simulink model "controller_observer_AllAgents_v5"  (Simulink 8.4)
settings: Solver=ode1  StartTime=0.0  StopTime=inf  FixedStep=60*1  RelTol=1e-3
values from neighbouring files (symbols this model uses):
  Cpw = 4180    [readepJSONout.m]
  m_max = 3    [DesignParameters.m]
  mc_MAX = 2    [DesignParameters.m]
  Tamb_additive_Sensor_fault_time = 60*60*24*(Tamb_day_ofthe_add_fault + Tamb_time_ofthe_add_fault)    (= 129600)    [faults_setting.m]
  Tamb_additive_Sensor_fault_value = 10    [faults_setting.m]
  Tamb_mult_Sensor_fault_time = 60*60*24*(Tamb_day_ofthe_mult_fault + Tamb_time_ofthe_mult_fault)    (= 129600)    [faults_setting.m]
  Tamb_mult_Sensor_fault_value = 1.1    [faults_setting.m]
  Ts = 3600/dat.Timestep.Timestep_0x20_1.number_of_timesteps_per_hour    [readepJSONout.m]
  Tst_C_additive_Sensor_fault_time = 60*60*24*(Tst_C_day_ofthe_add_fault + Tst_C_time_ofthe_add_fault)    (= 129600)    [faults_setting.m]
  Tst_C_additive_Sensor_fault_value = 10    [faults_setting.m]
  Tst_C_mult_Sensor_fault_time = 60*60*24*(Tst_C_day_ofthe_mult_fault + Tst_C_time_ofthe_mult_fault)    (= 129600)    [faults_setting.m]
  Tst_C_mult_Sensor_fault_value = 1.1    [faults_setting.m]
  Tst_H_additive_Sensor_fault_time = 60*60*24*(Tst_H_day_ofthe_add_fault + Tst_H_time_ofthe_add_fault)    (= 129600)    [faults_setting.m]
  Tst_H_additive_Sensor_fault_value = 10    [faults_setting.m]
  Tst_H_mult_Sensor_fault_time = 60*60*24*(Tst_H_day_ofthe_mult_fault + Tst_H_time_ofthe_mult_fault)    (= 129600)    [faults_setting.m]
  Tst_H_mult_Sensor_fault_value = 1.1    [faults_setting.m]
undefined symbols (not defined in the file or in its folder): building, COP, Ehp_max, fsa_E, fsa_ET, fsa_N, fsa_NT, fsa_W, fsa_WT, h_E, h_ET, h_N, h_NT, h_W, h_WT, K_NtoNT, K_NTtoN, Ke_E, Ke_ET, Ke_N, Ke_NT, Ke_WT, Km_E, Km_ET, Km_N, Km_NT, Km_W, Km_WT, Kout_N, Kout_NT, Kout_W, Kout_WT, Kst, Ksta, Kstcij, KstRef, Kw_E, Kw_ET, Kw_N, Kw_NT, Kw_W, Kw_WT
libraries: simulink x61
(part 16 of 36)

== system controller_observer_AllAgents_v5/Controller/Fault_mc_MainCorridor_Cooling ==
  In : Inport
  Add : Sum  Inputs=+++  SampleTime=Ts
  Add2 : Sum  Inputs=+-  SampleTime=Ts
  Clock : Clock
  Constant : Constant  Value=building.zone(17).mc_C_mult_Sensor_fault_time  SampleTime=Ts
  Constant2 : Constant  Value=building.zone(17).mc_C_incr_Sensor_fault_time  SampleTime=Ts
  Dot Product : DotProduct  SampleTime=Ts  InputSameDT=on  OutDataTypeStr=Inherit: Inherit via internal rule
  Fcn : Fcn  Expr=1-0.99^(u)  SampleTime=Ts
  Gain : Gain  Gain=building.zone(17).mc_C_mult_Sensor_fault_value  SampleTime=Ts
  Gain1 : Gain  Gain=building.zone(17).mc_C_incr_Sensor_fault_value  SampleTime=Ts
  Gain3 : Gain  Gain=1/Ts  SampleTime=Ts
  If : If  NumInputs=2  IfExpression=u1 >= u2  SampleTime=Ts
  If Action Subsystem : Subsystem  TreatAsAtomicUnit=on
  If Action Subsystem1 : Subsystem  TreatAsAtomicUnit=on
  Merge : Merge
  Step : Step  Time=building.zone(17).mc_C_additive_Sensor_fault_time  After=building.zone(17).mc_C_additive_Sensor_fault_value  SampleTime=Ts
  Step1 : Step  Time=building.zone(17).mc_C_incr_Sensor_fault_time  SampleTime=Ts
  Out : Outport
  wires:
  If/2 -> If Action Subsystem1/Action
  Add/1 -> Out/1
  Step/1 -> Add/1
  Merge/1 -> Add/2
  If/1 -> If Action Subsystem/Action
  If Action Subsystem/1(Out1) -> Merge/1
  In/1 -> If Action Subsystem1/1(In1), Gain/1
  If Action Subsystem1/1(Out1) -> Merge/2
  Gain/1 -> If Action Subsystem/1(In1)
  Constant/1 -> If/2
  Clock/1 -> Add2/1, If/1  "k"
  Dot Product/1 -> Fcn/1
  Step1/1 -> Dot Product/2
  Constant2/1 -> Add2/2
  Fcn/1 -> Gain1/1
  Add2/1 -> Gain3/1
  Gain3/1 -> Dot Product/1
  Gain1/1 -> Add/3

== system controller_observer_AllAgents_v5/Controller/Fault_mc_MainCorridor_Heating ==
  In : Inport
  Add : Sum  Inputs=+++  SampleTime=Ts
  Add2 : Sum  Inputs=+-  SampleTime=Ts
  Clock : Clock
  Constant : Constant  Value=building.zone(17).mc_H_mult_Sensor_fault_time  SampleTime=Ts
  Constant2 : Constant  Value=building.zone(17).mc_H_incr_Sensor_fault_time  SampleTime=Ts
  Dot Product : DotProduct  SampleTime=Ts  InputSameDT=on  OutDataTypeStr=Inherit: Inherit via internal rule
  Fcn : Fcn  Expr=1-0.99^(u)  SampleTime=Ts
  Gain : Gain  Gain=building.zone(17).mc_H_mult_Sensor_fault_value  SampleTime=Ts
  Gain1 : Gain  Gain=building.zone(17).mc_H_incr_Sensor_fault_value  SampleTime=Ts
  Gain3 : Gain  Gain=1/Ts  SampleTime=Ts
  If : If  NumInputs=2  IfExpression=u1 >= u2  SampleTime=Ts
  If Action Subsystem : Subsystem  TreatAsAtomicUnit=on
  If Action Subsystem1 : Subsystem  TreatAsAtomicUnit=on
  Merge : Merge
  Step : Step  Time=building.zone(17).mc_H_additive_Sensor_fault_time  After=building.zone(17).mc_H_additive_Sensor_fault_value  SampleTime=Ts
  Step1 : Step  Time=building.zone(17).mc_H_incr_Sensor_fault_time  SampleTime=Ts
  Out : Outport
  wires:
  If/2 -> If Action Subsystem1/Action
  Add/1 -> Out/1
  Step/1 -> Add/1
  Merge/1 -> Add/2
  If/1 -> If Action Subsystem/Action
  If Action Subsystem/1(Out1) -> Merge/1
  In/1 -> If Action Subsystem1/1(In1), Gain/1
  If Action Subsystem1/1(Out1) -> Merge/2
  Gain/1 -> If Action Subsystem/1(In1)
  Constant/1 -> If/2
  Clock/1 -> Add2/1, If/1  "k"
  Dot Product/1 -> Fcn/1
  Step1/1 -> Dot Product/2
  Constant2/1 -> Add2/2
  Fcn/1 -> Gain1/1
  Add2/1 -> Gain3/1
  Gain3/1 -> Dot Product/1
  Gain1/1 -> Add/3

== system controller_observer_AllAgents_v5/Controller/Fault_mc_Mech_Cooling ==
  In : Inport
  Add : Sum  Inputs=+++  SampleTime=Ts
  Add2 : Sum  Inputs=+-  SampleTime=Ts
  Clock : Clock
  Constant : Constant  Value=building.zone(18).mc_C_mult_Sensor_fault_time  SampleTime=Ts
  Constant2 : Constant  Value=building.zone(18).mc_C_incr_Sensor_fault_time  SampleTime=Ts
  Dot Product : DotProduct  SampleTime=Ts  InputSameDT=on  OutDataTypeStr=Inherit: Inherit via internal rule
  Fcn : Fcn  Expr=1-0.99^(u)  SampleTime=Ts
  Gain : Gain  Gain=building.zone(18).mc_C_mult_Sensor_fault_value  SampleTime=Ts
  Gain1 : Gain  Gain=building.zone(18).mc_C_incr_Sensor_fault_value  SampleTime=Ts
  Gain3 : Gain  Gain=1/Ts  SampleTime=Ts
  If : If  NumInputs=2  IfExpression=u1 >= u2  SampleTime=Ts
  If Action Subsystem : Subsystem  TreatAsAtomicUnit=on
  If Action Subsystem1 : Subsystem  TreatAsAtomicUnit=on
  Merge : Merge
  Step : Step  Time=building.zone(18).mc_C_additive_Sensor_fault_time  After=building.zone(18).mc_C_additive_Sensor_fault_value  SampleTime=Ts
  Step1 : Step  Time=building.zone(18).mc_C_incr_Sensor_fault_time  SampleTime=Ts
  Out : Outport
  wires:
  If/2 -> If Action Subsystem1/Action
  Add/1 -> Out/1
  Step/1 -> Add/1
  Merge/1 -> Add/2
  If/1 -> If Action Subsystem/Action
  If Action Subsystem/1(Out1) -> Merge/1
  In/1 -> If Action Subsystem1/1(In1), Gain/1
  If Action Subsystem1/1(Out1) -> Merge/2
  Gain/1 -> If Action Subsystem/1(In1)
  Constant/1 -> If/2
  Clock/1 -> Add2/1, If/1  "k"
  Dot Product/1 -> Fcn/1
  Step1/1 -> Dot Product/2
  Constant2/1 -> Add2/2
  Fcn/1 -> Gain1/1
  Add2/1 -> Gain3/1
  Gain3/1 -> Dot Product/1
  Gain1/1 -> Add/3

== system controller_observer_AllAgents_v5/Controller/Fault_mc_Mech_Heating ==
  In : Inport
  Add : Sum  Inputs=+++  SampleTime=Ts
  Add2 : Sum  Inputs=+-  SampleTime=Ts
  Clock : Clock
  Constant : Constant  Value=building.zone(18).mc_H_mult_Sensor_fault_time  SampleTime=Ts
  Constant2 : Constant  Value=building.zone(18).mc_H_incr_Sensor_fault_time  SampleTime=Ts
  Dot Product : DotProduct  SampleTime=Ts  InputSameDT=on  OutDataTypeStr=Inherit: Inherit via internal rule
  Fcn : Fcn  Expr=1-0.99^(u)  SampleTime=Ts
  Gain : Gain  Gain=building.zone(18).mc_H_mult_Sensor_fault_value  SampleTime=Ts
  Gain1 : Gain  Gain=building.zone(18).mc_H_incr_Sensor_fault_value  SampleTime=Ts
  Gain3 : Gain  Gain=1/Ts  SampleTime=Ts
  If : If  NumInputs=2  IfExpression=u1 >= u2  SampleTime=Ts
  If Action Subsystem : Subsystem  TreatAsAtomicUnit=on
  If Action Subsystem1 : Subsystem  TreatAsAtomicUnit=on
  Merge : Merge
  Step : Step  Time=building.zone(18).mc_H_additive_Sensor_fault_time  After=building.zone(18).mc_H_additive_Sensor_fault_value  SampleTime=Ts
  Step1 : Step  Time=building.zone(18).mc_H_incr_Sensor_fault_time  SampleTime=Ts
  Out : Outport
  wires:
  If/2 -> If Action Subsystem1/Action
  Add/1 -> Out/1
  Step/1 -> Add/1
  Merge/1 -> Add/2
  If/1 -> If Action Subsystem/Action
  If Action Subsystem/1(Out1) -> Merge/1
  In/1 -> If Action Subsystem1/1(In1), Gain/1
  If Action Subsystem1/1(Out1) -> Merge/2
  Gain/1 -> If Action Subsystem/1(In1)
  Constant/1 -> If/2
  Clock/1 -> Add2/1, If/1  "k"
  Dot Product/1 -> Fcn/1
  Step1/1 -> Dot Product/2
  Constant2/1 -> Add2/2
  Fcn/1 -> Gain1/1
  Add2/1 -> Gain3/1
  Gain3/1 -> Dot Product/1
  Gain1/1 -> Add/3

== system controller_observer_AllAgents_v5/Controller/Fault_mc_MultiClass1Pod1_Cooling ==
  In : Inport
  Add : Sum  Inputs=+++  SampleTime=Ts
  Add2 : Sum  Inputs=+-  SampleTime=Ts
  Clock : Clock
  Constant : Constant  Value=building.zone(19).mc_C_mult_Sensor_fault_time  SampleTime=Ts
  Constant2 : Constant  Value=building.zone(19).mc_C_incr_Sensor_fault_time  SampleTime=Ts
  Dot Product : DotProduct  SampleTime=Ts  InputSameDT=on  OutDataTypeStr=Inherit: Inherit via internal rule
  Fcn : Fcn  Expr=1-0.99^(u)  SampleTime=Ts
  Gain : Gain  Gain=building.zone(19).mc_C_mult_Sensor_fault_value  SampleTime=Ts
  Gain1 : Gain  Gain=building.zone(19).mc_C_incr_Sensor_fault_value  SampleTime=Ts
  Gain3 : Gain  Gain=1/Ts  SampleTime=Ts
  If : If  NumInputs=2  IfExpression=u1 >= u2  SampleTime=Ts
  If Action Subsystem : Subsystem  TreatAsAtomicUnit=on
  If Action Subsystem1 : Subsystem  TreatAsAtomicUnit=on
  Merge : Merge
  Step : Step  Time=building.zone(19).mc_C_additive_Sensor_fault_time  After=building.zone(19).mc_C_additive_Sensor_fault_value  SampleTime=Ts
  Step1 : Step  Time=building.zone(19).mc_C_incr_Sensor_fault_time  SampleTime=Ts
  Out : Outport
  wires:
  If/2 -> If Action Subsystem1/Action
  Add/1 -> Out/1
  Step/1 -> Add/1
  Merge/1 -> Add/2
  If/1 -> If Action Subsystem/Action
  If Action Subsystem/1(Out1) -> Merge/1
  In/1 -> If Action Subsystem1/1(In1), Gain/1
  If Action Subsystem1/1(Out1) -> Merge/2
  Gain/1 -> If Action Subsystem/1(In1)
  Constant/1 -> If/2
  Clock/1 -> Add2/1, If/1  "k"
  Dot Product/1 -> Fcn/1
  Step1/1 -> Dot Product/2
  Constant2/1 -> Add2/2
  Fcn/1 -> Gain1/1
  Add2/1 -> Gain3/1
  Gain3/1 -> Dot Product/1
  Gain1/1 -> Add/3

== system controller_observer_AllAgents_v5/Controller/Fault_mc_MultiClass1Pod1_Heating ==
  In : Inport
  Add : Sum  Inputs=+++  SampleTime=Ts
  Add2 : Sum  Inputs=+-  SampleTime=Ts
  Clock : Clock
  Constant : Constant  Value=building.zone(19).mc_H_mult_Sensor_fault_time  SampleTime=Ts
  Constant2 : Constant  Value=building.zone(19).mc_H_incr_Sensor_fault_time  SampleTime=Ts
  Dot Product : DotProduct  SampleTime=Ts  InputSameDT=on  OutDataTypeStr=Inherit: Inherit via internal rule
  Fcn : Fcn  Expr=1-0.99^(u)  SampleTime=Ts
  Gain : Gain  Gain=building.zone(19).mc_H_mult_Sensor_fault_value  SampleTime=Ts
  Gain1 : Gain  Gain=building.zone(19).mc_H_incr_Sensor_fault_value  SampleTime=Ts
  Gain3 : Gain  Gain=1/Ts  SampleTime=Ts
  If : If  NumInputs=2  IfExpression=u1 >= u2  SampleTime=Ts
  If Action Subsystem : Subsystem  TreatAsAtomicUnit=on
  If Action Subsystem1 : Subsystem  TreatAsAtomicUnit=on
  Merge : Merge
  Step : Step  Time=building.zone(19).mc_H_additive_Sensor_fault_time  After=building.zone(19).mc_H_additive_Sensor_fault_value  SampleTime=Ts
  Step1 : Step  Time=building.zone(19).mc_H_incr_Sensor_fault_time  SampleTime=Ts
  Out : Outport
  wires:
  If/2 -> If Action Subsystem1/Action
  Add/1 -> Out/1
  Step/1 -> Add/1
  Merge/1 -> Add/2
  If/1 -> If Action Subsystem/Action
  If Action Subsystem/1(Out1) -> Merge/1
  In/1 -> If Action Subsystem1/1(In1), Gain/1
  If Action Subsystem1/1(Out1) -> Merge/2
  Gain/1 -> If Action Subsystem/1(In1)
  Constant/1 -> If/2
  Clock/1 -> Add2/1, If/1  "k"
  Dot Product/1 -> Fcn/1
  Step1/1 -> Dot Product/2
  Constant2/1 -> Add2/2
  Fcn/1 -> Gain1/1
  Add2/1 -> Gain3/1
  Gain3/1 -> Dot Product/1
  Gain1/1 -> Add/3

== system controller_observer_AllAgents_v5/Controller/Fault_mc_MultiClass1Pod2_Cooling ==
  In : Inport
  Add : Sum  Inputs=+++  SampleTime=Ts
  Add2 : Sum  Inputs=+-  SampleTime=Ts
  Clock : Clock
  Constant : Constant  Value=building.zone(20).mc_C_mult_Sensor_fault_time  SampleTime=Ts
  Constant2 : Constant  Value=building.zone(20).mc_C_incr_Sensor_fault_time  SampleTime=Ts
  Dot Product : DotProduct  SampleTime=Ts  InputSameDT=on  OutDataTypeStr=Inherit: Inherit via internal rule
  Fcn : Fcn  Expr=1-0.99^(u)  SampleTime=Ts
  Gain : Gain  Gain=building.zone(20).mc_C_mult_Sensor_fault_value  SampleTime=Ts
  Gain1 : Gain  Gain=building.zone(20).mc_C_incr_Sensor_fault_value  SampleTime=Ts
  Gain3 : Gain  Gain=1/Ts  SampleTime=Ts
  If : If  NumInputs=2  IfExpression=u1 >= u2  SampleTime=Ts
  If Action Subsystem : Subsystem  TreatAsAtomicUnit=on
  If Action Subsystem1 : Subsystem  TreatAsAtomicUnit=on
  Merge : Merge
  Step : Step  Time=building.zone(20).mc_C_additive_Sensor_fault_time  After=building.zone(20).mc_C_additive_Sensor_fault_value  SampleTime=Ts
  Step1 : Step  Time=building.zone(20).mc_C_incr_Sensor_fault_time  SampleTime=Ts
  Out : Outport
  wires:
  If/2 -> If Action Subsystem1/Action
  Add/1 -> Out/1
  Step/1 -> Add/1
  Merge/1 -> Add/2
  If/1 -> If Action Subsystem/Action
  If Action Subsystem/1(Out1) -> Merge/1
  In/1 -> If Action Subsystem1/1(In1), Gain/1
  If Action Subsystem1/1(Out1) -> Merge/2
  Gain/1 -> If Action Subsystem/1(In1)
  Constant/1 -> If/2
  Clock/1 -> Add2/1, If/1  "k"
  Dot Product/1 -> Fcn/1
  Step1/1 -> Dot Product/2
  Constant2/1 -> Add2/2
  Fcn/1 -> Gain1/1
  Add2/1 -> Gain3/1
  Gain3/1 -> Dot Product/1
  Gain1/1 -> Add/3

== system controller_observer_AllAgents_v5/Controller/Fault_mc_MultiClass1Pod2_Heating ==
  In : Inport
  Add : Sum  Inputs=+++  SampleTime=Ts
  Add2 : Sum  Inputs=+-  SampleTime=Ts
  Clock : Clock
  Constant : Constant  Value=building.zone(20).mc_H_mult_Sensor_fault_time  SampleTime=Ts
  Constant2 : Constant  Value=building.zone(20).mc_H_incr_Sensor_fault_time  SampleTime=Ts
  Dot Product : DotProduct  SampleTime=Ts  InputSameDT=on  OutDataTypeStr=Inherit: Inherit via internal rule
  Fcn : Fcn  Expr=1-0.99^(u)  SampleTime=Ts
  Gain : Gain  Gain=building.zone(20).mc_H_mult_Sensor_fault_value  SampleTime=Ts
  Gain1 : Gain  Gain=building.zone(20).mc_H_incr_Sensor_fault_value  SampleTime=Ts
  Gain3 : Gain  Gain=1/Ts  SampleTime=Ts
  If : If  NumInputs=2  IfExpression=u1 >= u2  SampleTime=Ts
  If Action Subsystem : Subsystem  TreatAsAtomicUnit=on
  If Action Subsystem1 : Subsystem  TreatAsAtomicUnit=on
  Merge : Merge
  Step : Step  Time=building.zone(20).mc_H_additive_Sensor_fault_time  After=building.zone(20).mc_H_additive_Sensor_fault_value  SampleTime=Ts
  Step1 : Step  Time=building.zone(20).mc_H_incr_Sensor_fault_time  SampleTime=Ts
  Out : Outport
  wires:
  If/2 -> If Action Subsystem1/Action
  Add/1 -> Out/1
  Step/1 -> Add/1
  Merge/1 -> Add/2
  If/1 -> If Action Subsystem/Action
  If Action Subsystem/1(Out1) -> Merge/1
  In/1 -> If Action Subsystem1/1(In1), Gain/1
  If Action Subsystem1/1(Out1) -> Merge/2
  Gain/1 -> If Action Subsystem/1(In1)
  Constant/1 -> If/2
  Clock/1 -> Add2/1, If/1  "k"
  Dot Product/1 -> Fcn/1
  Step1/1 -> Dot Product/2
  Constant2/1 -> Add2/2
  Fcn/1 -> Gain1/1
  Add2/1 -> Gain3/1
  Gain3/1 -> Dot Product/1
  Gain1/1 -> Add/3

== system controller_observer_AllAgents_v5/Controller/Fault_mc_MultiClass1Pod3_Cooling ==
  In : Inport
  Add : Sum  Inputs=+++  SampleTime=Ts
  Add2 : Sum  Inputs=+-  SampleTime=Ts
  Clock : Clock
  Constant : Constant  Value=building.zone(21).mc_C_mult_Sensor_fault_time  SampleTime=Ts
  Constant2 : Constant  Value=building.zone(21).mc_C_incr_Sensor_fault_time  SampleTime=Ts
  Dot Product : DotProduct  SampleTime=Ts  InputSameDT=on  OutDataTypeStr=Inherit: Inherit via internal rule
  Fcn : Fcn  Expr=1-0.99^(u)  SampleTime=Ts
  Gain : Gain  Gain=building.zone(21).mc_C_mult_Sensor_fault_value  SampleTime=Ts
  Gain1 : Gain  Gain=building.zone(21).mc_C_incr_Sensor_fault_value  SampleTime=Ts
  Gain3 : Gain  Gain=1/Ts  SampleTime=Ts
  If : If  NumInputs=2  IfExpression=u1 >= u2  SampleTime=Ts
  If Action Subsystem : Subsystem  TreatAsAtomicUnit=on
  If Action Subsystem1 : Subsystem  TreatAsAtomicUnit=on
  Merge : Merge
  Step : Step  Time=building.zone(21).mc_C_additive_Sensor_fault_time  After=building.zone(21).mc_C_additive_Sensor_fault_value  SampleTime=Ts
  Step1 : Step  Time=building.zone(21).mc_C_incr_Sensor_fault_time  SampleTime=Ts
  Out : Outport
  wires:
  If/2 -> If Action Subsystem1/Action
  Add/1 -> Out/1
  Step/1 -> Add/1
  Merge/1 -> Add/2
  If/1 -> If Action Subsystem/Action
  If Action Subsystem/1(Out1) -> Merge/1
  In/1 -> If Action Subsystem1/1(In1), Gain/1
  If Action Subsystem1/1(Out1) -> Merge/2
  Gain/1 -> If Action Subsystem/1(In1)
  Constant/1 -> If/2
  Clock/1 -> Add2/1, If/1  "k"
  Dot Product/1 -> Fcn/1
  Step1/1 -> Dot Product/2
  Constant2/1 -> Add2/2
  Fcn/1 -> Gain1/1
  Add2/1 -> Gain3/1
  Gain3/1 -> Dot Product/1
  Gain1/1 -> Add/3

== system controller_observer_AllAgents_v5/Controller/Fault_mc_MultiClass1Pod3_Heating ==
  In : Inport
  Add : Sum  Inputs=+++  SampleTime=Ts
  Add2 : Sum  Inputs=+-  SampleTime=Ts
  Clock : Clock
  Constant : Constant  Value=building.zone(21).mc_H_mult_Sensor_fault_time  SampleTime=Ts
  Constant2 : Constant  Value=building.zone(21).mc_H_incr_Sensor_fault_time  SampleTime=Ts
  Dot Product : DotProduct  SampleTime=Ts  InputSameDT=on  OutDataTypeStr=Inherit: Inherit via internal rule
  Fcn : Fcn  Expr=1-0.99^(u)  SampleTime=Ts
  Gain : Gain  Gain=building.zone(21).mc_H_mult_Sensor_fault_value  SampleTime=Ts
  Gain1 : Gain  Gain=building.zone(21).mc_H_incr_Sensor_fault_value  SampleTime=Ts
  Gain3 : Gain  Gain=1/Ts  SampleTime=Ts
  If : If  NumInputs=2  IfExpression=u1 >= u2  SampleTime=Ts
  If Action Subsystem : Subsystem  TreatAsAtomicUnit=on
  If Action Subsystem1 : Subsystem  TreatAsAtomicUnit=on
  Merge : Merge
  Step : Step  Time=building.zone(21).mc_H_additive_Sensor_fault_time  After=building.zone(21).mc_H_additive_Sensor_fault_value  SampleTime=Ts
  Step1 : Step  Time=building.zone(21).mc_H_incr_Sensor_fault_time  SampleTime=Ts
  Out : Outport
  wires:
  If/2 -> If Action Subsystem1/Action
  Add/1 -> Out/1
  Step/1 -> Add/1
  Merge/1 -> Add/2
  If/1 -> If Action Subsystem/Action
  If Action Subsystem/1(Out1) -> Merge/1
  In/1 -> If Action Subsystem1/1(In1), Gain/1
  If Action Subsystem1/1(Out1) -> Merge/2
  Gain/1 -> If Action Subsystem/1(In1)
  Constant/1 -> If/2
  Clock/1 -> Add2/1, If/1  "k"
  Dot Product/1 -> Fcn/1
  Step1/1 -> Dot Product/2
  Constant2/1 -> Add2/2
  Fcn/1 -> Gain1/1
  Add2/1 -> Gain3/1
  Gain3/1 -> Dot Product/1
  Gain1/1 -> Add/3

== system controller_observer_AllAgents_v5/Controller/Fault_mc_MultiClass2Pod1_Cooling ==
  In : Inport
  Add : Sum  Inputs=+++  SampleTime=Ts
  Add2 : Sum  Inputs=+-  SampleTime=Ts
  Clock : Clock
  Constant : Constant  Value=building.zone(22).mc_C_mult_Sensor_fault_time  SampleTime=Ts
  Constant2 : Constant  Value=building.zone(22).mc_C_incr_Sensor_fault_time  SampleTime=Ts
  Dot Product : DotProduct  SampleTime=Ts  InputSameDT=on  OutDataTypeStr=Inherit: Inherit via internal rule
  Fcn : Fcn  Expr=1-0.99^(u)  SampleTime=Ts
  Gain : Gain  Gain=building.zone(22).mc_C_mult_Sensor_fault_value  SampleTime=Ts
  Gain1 : Gain  Gain=building.zone(22).mc_C_incr_Sensor_fault_value  SampleTime=Ts
  Gain3 : Gain  Gain=1/Ts  SampleTime=Ts
  If : If  NumInputs=2  IfExpression=u1 >= u2  SampleTime=Ts
  If Action Subsystem : Subsystem  TreatAsAtomicUnit=on
  If Action Subsystem1 : Subsystem  TreatAsAtomicUnit=on
  Merge : Merge
  Step : Step  Time=building.zone(22).mc_C_additive_Sensor_fault_time  After=building.zone(22).mc_C_additive_Sensor_fault_value  SampleTime=Ts
  Step1 : Step  Time=building.zone(22).mc_C_incr_Sensor_fault_time  SampleTime=Ts
  Out : Outport
  wires:
  If/2 -> If Action Subsystem1/Action
  Add/1 -> Out/1
  Step/1 -> Add/1
  Merge/1 -> Add/2
  If/1 -> If Action Subsystem/Action
  If Action Subsystem/1(Out1) -> Merge/1
  In/1 -> If Action Subsystem1/1(In1), Gain/1
  If Action Subsystem1/1(Out1) -> Merge/2
  Gain/1 -> If Action Subsystem/1(In1)
  Constant/1 -> If/2
  Clock/1 -> Add2/1, If/1  "k"
  Dot Product/1 -> Fcn/1
  Step1/1 -> Dot Product/2
  Constant2/1 -> Add2/2
  Fcn/1 -> Gain1/1
  Add2/1 -> Gain3/1
  Gain3/1 -> Dot Product/1
  Gain1/1 -> Add/3

== system controller_observer_AllAgents_v5/Controller/Fault_mc_MultiClass2Pod1_Heating ==
  In : Inport
  Add : Sum  Inputs=+++  SampleTime=Ts
  Add2 : Sum  Inputs=+-  SampleTime=Ts
  Clock : Clock
  Constant : Constant  Value=building.zone(22).mc_H_mult_Sensor_fault_time  SampleTime=Ts
  Constant2 : Constant  Value=building.zone(22).mc_H_incr_Sensor_fault_time  SampleTime=Ts
  Dot Product : DotProduct  SampleTime=Ts  InputSameDT=on  OutDataTypeStr=Inherit: Inherit via internal rule
  Fcn : Fcn  Expr=1-0.99^(u)  SampleTime=Ts
  Gain : Gain  Gain=building.zone(22).mc_H_mult_Sensor_fault_value  SampleTime=Ts
  Gain1 : Gain  Gain=building.zone(22).mc_H_incr_Sensor_fault_value  SampleTime=Ts
  Gain3 : Gain  Gain=1/Ts  SampleTime=Ts
  If : If  NumInputs=2  IfExpression=u1 >= u2  SampleTime=Ts
  If Action Subsystem : Subsystem  TreatAsAtomicUnit=on
  If Action Subsystem1 : Subsystem  TreatAsAtomicUnit=on
  Merge : Merge
  Step : Step  Time=building.zone(22).mc_H_additive_Sensor_fault_time  After=building.zone(22).mc_H_additive_Sensor_fault_value  SampleTime=Ts
  Step1 : Step  Time=building.zone(22).mc_H_incr_Sensor_fault_time  SampleTime=Ts
  Out : Outport
  wires:
  If/2 -> If Action Subsystem1/Action
  Add/1 -> Out/1
  Step/1 -> Add/1
  Merge/1 -> Add/2
  If/1 -> If Action Subsystem/Action
  If Action Subsystem/1(Out1) -> Merge/1
  In/1 -> If Action Subsystem1/1(In1), Gain/1
  If Action Subsystem1/1(Out1) -> Merge/2
  Gain/1 -> If Action Subsystem/1(In1)
  Constant/1 -> If/2
  Clock/1 -> Add2/1, If/1  "k"
  Dot Product/1 -> Fcn/1
  Step1/1 -> Dot Product/2
  Constant2/1 -> Add2/2
  Fcn/1 -> Gain1/1
  Add2/1 -> Gain3/1
  Gain3/1 -> Dot Product/1
  Gain1/1 -> Add/3

== system controller_observer_AllAgents_v5/Controller/Fault_mc_MultiClass2Pod2_Cooling ==
  In : Inport
  Add : Sum  Inputs=+++  SampleTime=Ts
  Add2 : Sum  Inputs=+-  SampleTime=Ts
  Clock : Clock
  Constant : Constant  Value=building.zone(23).mc_C_mult_Sensor_fault_time  SampleTime=Ts
  Constant2 : Constant  Value=building.zone(23).mc_C_incr_Sensor_fault_time  SampleTime=Ts
  Dot Product : DotProduct  SampleTime=Ts  InputSameDT=on  OutDataTypeStr=Inherit: Inherit via internal rule
  Fcn : Fcn  Expr=1-0.99^(u)  SampleTime=Ts
  Gain : Gain  Gain=building.zone(23).mc_C_mult_Sensor_fault_value  SampleTime=Ts
  Gain1 : Gain  Gain=building.zone(23).mc_C_incr_Sensor_fault_value  SampleTime=Ts
  Gain3 : Gain  Gain=1/Ts  SampleTime=Ts
  If : If  NumInputs=2  IfExpression=u1 >= u2  SampleTime=Ts
  If Action Subsystem : Subsystem  TreatAsAtomicUnit=on
  If Action Subsystem1 : Subsystem  TreatAsAtomicUnit=on
  Merge : Merge
  Step : Step  Time=building.zone(23).mc_C_additive_Sensor_fault_time  After=building.zone(23).mc_C_additive_Sensor_fault_value  SampleTime=Ts
  Step1 : Step  Time=building.zone(23).mc_C_incr_Sensor_fault_time  SampleTime=Ts
  Out : Outport
  wires:
  If/2 -> If Action Subsystem1/Action
  Add/1 -> Out/1
  Step/1 -> Add/1
  Merge/1 -> Add/2
  If/1 -> If Action Subsystem/Action
  If Action Subsystem/1(Out1) -> Merge/1
  In/1 -> If Action Subsystem1/1(In1), Gain/1
  If Action Subsystem1/1(Out1) -> Merge/2
  Gain/1 -> If Action Subsystem/1(In1)
  Constant/1 -> If/2
  Clock/1 -> Add2/1, If/1  "k"
  Dot Product/1 -> Fcn/1
  Step1/1 -> Dot Product/2
  Constant2/1 -> Add2/2
  Fcn/1 -> Gain1/1
  Add2/1 -> Gain3/1
  Gain3/1 -> Dot Product/1
  Gain1/1 -> Add/3

== system controller_observer_AllAgents_v5/Controller/Fault_mc_MultiClass2Pod2_Heating ==
  In : Inport
  Add : Sum  Inputs=+++  SampleTime=Ts
  Add2 : Sum  Inputs=+-  SampleTime=Ts
  Clock : Clock
  Constant : Constant  Value=building.zone(23).mc_H_mult_Sensor_fault_time  SampleTime=Ts
  Constant2 : Constant  Value=building.zone(23).mc_H_incr_Sensor_fault_time  SampleTime=Ts
  Dot Product : DotProduct  SampleTime=Ts  InputSameDT=on  OutDataTypeStr=Inherit: Inherit via internal rule
  Fcn : Fcn  Expr=1-0.99^(u)  SampleTime=Ts
  Gain : Gain  Gain=building.zone(23).mc_H_mult_Sensor_fault_value  SampleTime=Ts
  Gain1 : Gain  Gain=building.zone(23).mc_H_incr_Sensor_fault_value  SampleTime=Ts
  Gain3 : Gain  Gain=1/Ts  SampleTime=Ts
  If : If  NumInputs=2  IfExpression=u1 >= u2  SampleTime=Ts
  If Action Subsystem : Subsystem  TreatAsAtomicUnit=on
  If Action Subsystem1 : Subsystem  TreatAsAtomicUnit=on
  Merge : Merge
  Step : Step  Time=building.zone(23).mc_H_additive_Sensor_fault_time  After=building.zone(23).mc_H_additive_Sensor_fault_value  SampleTime=Ts
  Step1 : Step  Time=building.zone(23).mc_H_incr_Sensor_fault_time  SampleTime=Ts
  Out : Outport
  wires:
  If/2 -> If Action Subsystem1/Action
  Add/1 -> Out/1
  Step/1 -> Add/1
  Merge/1 -> Add/2
  If/1 -> If Action Subsystem/Action
  If Action Subsystem/1(Out1) -> Merge/1
  In/1 -> If Action Subsystem1/1(In1), Gain/1
  If Action Subsystem1/1(Out1) -> Merge/2
  Gain/1 -> If Action Subsystem/1(In1)
  Constant/1 -> If/2
  Clock/1 -> Add2/1, If/1  "k"
  Dot Product/1 -> Fcn/1
  Step1/1 -> Dot Product/2
  Constant2/1 -> Add2/2
  Fcn/1 -> Gain1/1
  Add2/1 -> Gain3/1
  Gain3/1 -> Dot Product/1
  Gain1/1 -> Add/3

== system controller_observer_AllAgents_v5/Controller/Fault_mc_MultiClass2Pod3_Cooling ==
  In : Inport
  Add : Sum  Inputs=+++  SampleTime=Ts
  Add2 : Sum  Inputs=+-  SampleTime=Ts
  Clock : Clock
  Constant : Constant  Value=building.zone(24).mc_C_mult_Sensor_fault_time  SampleTime=Ts
  Constant2 : Constant  Value=building.zone(24).mc_C_incr_Sensor_fault_time  SampleTime=Ts
  Dot Product : DotProduct  SampleTime=Ts  InputSameDT=on  OutDataTypeStr=Inherit: Inherit via internal rule
  Fcn : Fcn  Expr=1-0.99^(u)  SampleTime=Ts
  Gain : Gain  Gain=building.zone(24).mc_C_mult_Sensor_fault_value  SampleTime=Ts
  Gain1 : Gain  Gain=building.zone(24).mc_C_incr_Sensor_fault_value  SampleTime=Ts
  Gain3 : Gain  Gain=1/Ts  SampleTime=Ts
  If : If  NumInputs=2  IfExpression=u1 >= u2  SampleTime=Ts
  If Action Subsystem : Subsystem  TreatAsAtomicUnit=on
  If Action Subsystem1 : Subsystem  TreatAsAtomicUnit=on
  Merge : Merge
  Step : Step  Time=building.zone(24).mc_C_additive_Sensor_fault_time  After=building.zone(24).mc_C_additive_Sensor_fault_value  SampleTime=Ts
  Step1 : Step  Time=building.zone(24).mc_C_incr_Sensor_fault_time  SampleTime=Ts
  Out : Outport
  wires:
  If/2 -> If Action Subsystem1/Action
  Add/1 -> Out/1
  Step/1 -> Add/1
  Merge/1 -> Add/2
  If/1 -> If Action Subsystem/Action
  If Action Subsystem/1(Out1) -> Merge/1
  In/1 -> If Action Subsystem1/1(In1), Gain/1
  If Action Subsystem1/1(Out1) -> Merge/2
  Gain/1 -> If Action Subsystem/1(In1)
  Constant/1 -> If/2
  Clock/1 -> Add2/1, If/1  "k"
  Dot Product/1 -> Fcn/1
  Step1/1 -> Dot Product/2
  Constant2/1 -> Add2/2
  Fcn/1 -> Gain1/1
  Add2/1 -> Gain3/1
  Gain3/1 -> Dot Product/1
  Gain1/1 -> Add/3

== system controller_observer_AllAgents_v5/Controller/Fault_mc_MultiClass2Pod3_Heating ==
  In : Inport
  Add : Sum  Inputs=+++  SampleTime=Ts
  Add2 : Sum  Inputs=+-  SampleTime=Ts
  Clock : Clock
  Constant : Constant  Value=building.zone(24).mc_H_mult_Sensor_fault_time  SampleTime=Ts
  Constant2 : Constant  Value=building.zone(24).mc_H_incr_Sensor_fault_time  SampleTime=Ts
  Dot Product : DotProduct  SampleTime=Ts  InputSameDT=on  OutDataTypeStr=Inherit: Inherit via internal rule
  Fcn : Fcn  Expr=1-0.99^(u)  SampleTime=Ts
  Gain : Gain  Gain=building.zone(24).mc_H_mult_Sensor_fault_value  SampleTime=Ts
  Gain1 : Gain  Gain=building.zone(24).mc_H_incr_Sensor_fault_value  SampleTime=Ts
  Gain3 : Gain  Gain=1/Ts  SampleTime=Ts
  If : If  NumInputs=2  IfExpression=u1 >= u2  SampleTime=Ts
  If Action Subsystem : Subsystem  TreatAsAtomicUnit=on
  If Action Subsystem1 : Subsystem  TreatAsAtomicUnit=on
  Merge : Merge
  Step : Step  Time=building.zone(24).mc_H_additive_Sensor_fault_time  After=building.zone(24).mc_H_additive_Sensor_fault_value  SampleTime=Ts
  Step1 : Step  Time=building.zone(24).mc_H_incr_Sensor_fault_time  SampleTime=Ts
  Out : Outport
  wires:
  If/2 -> If Action Subsystem1/Action
  Add/1 -> Out/1
  Step/1 -> Add/1
  Merge/1 -> Add/2
  If/1 -> If Action Subsystem/Action
  If Action Subsystem/1(Out1) -> Merge/1
  In/1 -> If Action Subsystem1/1(In1), Gain/1
  If Action Subsystem1/1(Out1) -> Merge/2
  Gain/1 -> If Action Subsystem/1(In1)
  Constant/1 -> If/2
  Clock/1 -> Add2/1, If/1  "k"
  Dot Product/1 -> Fcn/1
  Step1/1 -> Dot Product/2
  Constant2/1 -> Add2/2
  Fcn/1 -> Gain1/1
  Add2/1 -> Gain3/1
  Gain3/1 -> Dot Product/1
  Gain1/1 -> Add/3

== system controller_observer_AllAgents_v5/Controller/Fault_mc_Offices_Cooling ==
  In : Inport
  Add : Sum  Inputs=+++  SampleTime=Ts
  Add2 : Sum  Inputs=+-  SampleTime=Ts
  Clock : Clock
  Constant : Constant  Value=building.zone(25).mc_C_mult_Sensor_fault_time  SampleTime=Ts
  Constant2 : Constant  Value=building.zone(25).mc_C_incr_Sensor_fault_time  SampleTime=Ts
  Dot Product : DotProduct  SampleTime=Ts  InputSameDT=on  OutDataTypeStr=Inherit: Inherit via internal rule
  Fcn : Fcn  Expr=1-0.99^(u)  SampleTime=Ts
  Gain : Gain  Gain=building.zone(25).mc_C_mult_Sensor_fault_value  SampleTime=Ts
  Gain1 : Gain  Gain=building.zone(25).mc_C_incr_Sensor_fault_value  SampleTime=Ts
  Gain3 : Gain  Gain=1/Ts  SampleTime=Ts
  If : If  NumInputs=2  IfExpression=u1 >= u2  SampleTime=Ts
  If Action Subsystem : Subsystem  TreatAsAtomicUnit=on
  If Action Subsystem1 : Subsystem  TreatAsAtomicUnit=on
  Merge : Merge
  Step : Step  Time=building.zone(25).mc_C_additive_Sensor_fault_time  After=building.zone(25).mc_C_additive_Sensor_fault_value  SampleTime=Ts
  Step1 : Step  Time=building.zone(25).mc_C_incr_Sensor_fault_time  SampleTime=Ts
  Out : Outport
  wires:
  If/2 -> If Action Subsystem1/Action
  Add/1 -> Out/1
  Step/1 -> Add/1
  Merge/1 -> Add/2
  If/1 -> If Action Subsystem/Action
  If Action Subsystem/1(Out1) -> Merge/1
  In/1 -> If Action Subsystem1/1(In1), Gain/1
  If Action Subsystem1/1(Out1) -> Merge/2
  Gain/1 -> If Action Subsystem/1(In1)
  Constant/1 -> If/2
  Clock/1 -> Add2/1, If/1  "k"
  Dot Product/1 -> Fcn/1
  Step1/1 -> Dot Product/2
  Constant2/1 -> Add2/2
  Fcn/1 -> Gain1/1
  Add2/1 -> Gain3/1
  Gain3/1 -> Dot Product/1
  Gain1/1 -> Add/3

== system controller_observer_AllAgents_v5/Controller/Fault_mc_Offices_Heating ==
  In : Inport
  Add : Sum  Inputs=+++  SampleTime=Ts
  Add2 : Sum  Inputs=+-  SampleTime=Ts
  Clock : Clock
  Constant : Constant  Value=building.zone(25).mc_H_mult_Sensor_fault_time  SampleTime=Ts
  Constant2 : Constant  Value=building.zone(25).mc_H_incr_Sensor_fault_time  SampleTime=Ts
  Dot Product : DotProduct  SampleTime=Ts  InputSameDT=on  OutDataTypeStr=Inherit: Inherit via internal rule
  Fcn : Fcn  Expr=1-0.99^(u)  SampleTime=Ts
  Gain : Gain  Gain=building.zone(25).mc_H_mult_Sensor_fault_value  SampleTime=Ts
  Gain1 : Gain  Gain=building.zone(25).mc_H_incr_Sensor_fault_value  SampleTime=Ts
  Gain3 : Gain  Gain=1/Ts  SampleTime=Ts
  If : If  NumInputs=2  IfExpression=u1 >= u2  SampleTime=Ts
  If Action Subsystem : Subsystem  TreatAsAtomicUnit=on
  If Action Subsystem1 : Subsystem  TreatAsAtomicUnit=on
  Merge : Merge
  Step : Step  Time=building.zone(25).mc_H_additive_Sensor_fault_time  After=building.zone(25).mc_H_additive_Sensor_fault_value  SampleTime=Ts
  Step1 : Step  Time=building.zone(25).mc_H_incr_Sensor_fault_time  SampleTime=Ts
  Out : Outport
  wires:
  If/2 -> If Action Subsystem1/Action
  Add/1 -> Out/1
  Step/1 -> Add/1
  Merge/1 -> Add/2
  If/1 -> If Action Subsystem/Action
  If Action Subsystem/1(Out1) -> Merge/1
  In/1 -> If Action Subsystem1/1(In1), Gain/1
  If Action Subsystem1/1(Out1) -> Merge/2
  Gain/1 -> If Action Subsystem/1(In1)
  Constant/1 -> If/2
  Clock/1 -> Add2/1, If/1  "k"
  Dot Product/1 -> Fcn/1
  Step1/1 -> Dot Product/2
  Constant2/1 -> Add2/2
  Fcn/1 -> Gain1/1
  Add2/1 -> Gain3/1
  Gain3/1 -> Dot Product/1
  Gain1/1 -> Add/3

== system controller_observer_AllAgents_v5/Controller/Gym_Agent ==
  In1 : Inport
  Enable : EnablePort  StatesWhenEnabling=reset  PropagateVarSize=During execution
  Abs{,1..3} (4 alike) : Abs
  Cc_C : Constant  Value=[building.zone(13).C_c_C]  SampleTime=Ts  FramePeriod=Ts
  CoilGains : Constant  Value=[building.zone(13).A_c_C]  SampleTime=Ts  FramePeriod=Ts
  CoilGains1 : Constant  Value=[building.zone(13).B_c_C]  SampleTime=Ts  FramePeriod=Ts
  CoilGains2 : Constant  Value=[building.zone(13).B_c_C*building.zone(13).Df_C]  SampleTime=Ts  FramePeriod=Ts
  CoilGains3 : Constant  Value=[building.zone(13).B_c_C*building.zone(13).Damb_C]  SampleTime=Ts  FramePeriod=Ts
  CoilGains4 : Constant  Value=[building.zone(13).B_c_C*building.zone(13).Dma_C]  SampleTime=Ts  FramePeriod=Ts
  CoilGains5 : Constant  Value=[building.zone(13).L_c_C]  SampleTime=Ts  FramePeriod=Ts
  Constant{,3,5,7..8,10,12} (7 alike) : Constant  SampleTime=Ts
  Constant{1,4,6,11} (4 alike) : Constant  Value=0  SampleTime=Ts
  Constant2 : Constant  Value=0
  Csa : Constant  Value=[building.zone(13).C_sa_C]  SampleTime=Ts  FramePeriod=Ts
  Demux : Demux  Outputs=[1 1 1 1 1 1 1 numel(building.zone(13).neighbour) 1 1]
  Detection_coil : ToFile  Filename=C:\Users\papad\Dropbox\Complete_building_simulator_Observers\Results_fz4_sensor\D_c_C_Gym.mat  SaveFormat=Timeseries  SampleTime=Ts
  Detection_hcoil : ToFile  Filename=C:\Users\papad\Dropbox\Complete_building_simulator_Observers\Results_fz4_sensor\D_c_H_Gym.mat  SaveFormat=Timeseries  SampleTime=Ts
  Detection_sa : ToFile  Filename=C:\Users\papad\Dropbox\Complete_building_simulator_Observers\Results_fz4_sensor\D_saC_Gym.mat  SaveFormat=Timeseries  SampleTime=Ts
  Detection_zone : ToFile  Filename=C:\Users\papad\Dropbox\Complete_building_simulator_Observers\Results_fz4_sensor\D_zC_Gym.mat  SaveFormat=Timeseries  SampleTime=Ts
  Dot Product{1..5,12,16} (7 alike) : DotProduct  SampleTime=Ts  InputSameDT=on  OutDataTypeStr=Inherit: Inherit via internal rule
  Enabled Subsystem : Subsystem  TreatAsAtomicUnit=on
  Enabled Subsystem1 : Subsystem  TreatAsAtomicUnit=on
  Enabled Subsystem2 : Subsystem  TreatAsAtomicUnit=on
  Enabled Subsystem3 : Subsystem  TreatAsAtomicUnit=on
  HCoilGains : Constant  Value=[building.zone(13).A_c_H]  SampleTime=Ts  FramePeriod=Ts
  HCoilGains1 : Constant  Value=[building.zone(13).B_c_H]  SampleTime=Ts  FramePeriod=Ts
  HCoilGains2 : Constant  Value=[building.zone(13).B_c_H*building.zone(13).Dfc_H]  SampleTime=Ts  FramePeriod=Ts
  HCoilGains3 : Constant  Value=[building.zone(13).B_c_H*building.zone(13).Dambc_H]  SampleTime=Ts  FramePeriod=Ts
  HCoilGains4 : Constant  Value=[building.zone(13).B_c_H*building.zone(13).Dmac_H]  SampleTime=Ts  FramePeriod=Ts
  HCoilGains5 : Constant  Value=[building.zone(13).L_c_H]  SampleTime=Ts  FramePeriod=Ts
  HCoilGains6 : Constant  Value=[building.zone(18).B_c_H]  SampleTime=Ts  FramePeriod=Ts
  Hc_H : Constant  Value=[building.zone(13).C_c_H]  SampleTime=Ts  FramePeriod=Ts
  Hc_H1 : Constant  Value=transpose([building.zone(13).C_c_H])  SampleTime=Ts  FramePeriod=Ts
  Hc_H2 : Constant  Value=transpose([0 1 0 0])  SampleTime=Ts  FramePeriod=Ts
  If Action Subsystem1 : Subsystem  TreatAsAtomicUnit=on
  If Action Subsystem2 : Subsystem  TreatAsAtomicUnit=on
  If Action Subsystem3 : Subsystem  TreatAsAtomicUnit=on
  If Action Subsystem4 : Subsystem  TreatAsAtomicUnit=on
  If Action Subsystem5 : Subsystem  TreatAsAtomicUnit=on
  If Action Subsystem6 : Subsystem  TreatAsAtomicUnit=on
  If Action Subsystem7 : Subsystem  TreatAsAtomicUnit=on
  If Action Subsystem8 : Subsystem  TreatAsAtomicUnit=on
  If{1..4} (4 alike) : If  NumInputs=2  IfExpression=u1 > u2  SampleTime=Ts
  Merge{,1..3} (4 alike) : Merge  InitialOutput=[0]
  Mux : Mux  Inputs=2
  Mux3 : Mux  Inputs=2
  Mux4 : Mux  Inputs=[1 1 numel(building.zone(13).neighbour) 1 1]
  Product{,1..20} (21 alike) : Product  Multiplication=Matrix(*)
  Saturation : Saturate  UpperLimit=mc_MAX  LowerLimit=0  LinearizeAsGain=off  SampleTime=Ts
  Saturation1 : Saturate  UpperLimit=mc_MAX  LowerLimit=0  LinearizeAsGain=off  SampleTime=Ts
  Subtract{1,4..5,7..10} (7 alike) : Sum  Inputs=+-  SampleTime=Ts
  Sum : Sum  Inputs=++++++  SampleTime=Ts
  Sum1 : Sum  Inputs=++++++  SampleTime=Ts
  Sum2 : Sum  Inputs=+++++++  SampleTime=Ts
  SupplyGains : Constant  Value=[building.zone(13).A_sa_C]  SampleTime=Ts  FramePeriod=Ts
  SupplyGains1 : Constant  Value=[building.zone(13).B_sa_C]  SampleTime=Ts  FramePeriod=Ts
  SupplyGains2 : Constant  Value=[building.zone(13).B_sa_C*building.zone(13).Df_SA]  SampleTime=Ts  FramePeriod=Ts
  SupplyGains3 : Constant  Value=[building.zone(13).B_sa_C*building.zone(13).Damb_SA]  SampleTime=Ts  FramePeriod=Ts
  SupplyGains4 : Constant  Value=[building.zone(13).B_sa_C*building.zone(13).Dma_SA]  SampleTime=Ts  FramePeriod=Ts
  SupplyGains5 : Constant  Value=[building.zone(13).L_sa_C]  SampleTime=Ts  FramePeriod=Ts
  Tchat_mat : ToFile  Filename=C:\Users\papad\Dropbox\Complete_building_simulator_Observers\Results_fz4_sensor\Tchat_Gym.mat  SaveFormat=Timeseries  SampleTime=Ts
  Thchat_mat : ToFile  Filename=C:\Users\papad\Dropbox\Complete_building_simulator_Observers\Results_fz4_sensor\TchatH_Gym.mat  SaveFormat=Timeseries  SampleTime=Ts
  Threshold_coil : Subsystem
  Threshold_hcoil : Subsystem
  Threshold_sa : Subsystem
  Threshold_zone : Subsystem
  Tsahat_mat : ToFile  Filename=C:\Users\papad\Dropbox\Complete_building_simulator_Observers\Results_fz4_sensor\Tsahat_Gym.mat  SaveFormat=Timeseries  SampleTime=Ts
  Tzhat_mat : ToFile  Filename=C:\Users\papad\Dropbox\Complete_building_simulator_Observers\Results_fz4_sensor\Tzhat_Gym.mat  SaveFormat=Timeseries  SampleTime=Ts
  Unit Delay1 : UnitDelay  InitialCondition=transpose([0 0 0 0])  SampleTime=Ts
  Unit Delay7 : UnitDelay  InitialCondition=[0 0]'  SampleTime=Ts
  Unit Delay8 : UnitDelay  InitialCondition=[0 0]'  SampleTime=Ts
  Unit Delay9 : UnitDelay  SampleTime=Ts
  ZoneGains : Constant  Value=[building.zone(13).A_z; building.zone(13).B_z; building.zone(13).B_z*transpose(building.zone(13).Kij); building.zone(13).B_z*building.zone(13).Kza; building.zone(13).L]  SampleTime=Ts  FramePeriod=Ts
  residual_coil : ToFile  Filename=C:\Users\papad\Dropbox\Complete_building_simulator_Observers\Results_fz4_sensor\Res_c_C_Gym.mat  SaveFormat=Timeseries  SampleTime=Ts
  residual_hcoil : ToFile  Filename=C:\Users\papad\Dropbox\Complete_building_simulator_Observers\Results_fz4_sensor\Res_c_H_Gym.mat  SaveFormat=Timeseries  SampleTime=Ts
  residual_sa : ToFile  Filename=C:\Users\papad\Dropbox\Complete_building_simulator_Observers\Results_fz4_sensor\Res_saC_Gym.mat  SaveFormat=Timeseries  SampleTime=Ts
  residual_zone : ToFile  Filename=C:\Users\papad\Dropbox\Complete_building_simulator_Observers\Results_fz4_sensor\Res_zC_Gym.mat  SaveFormat=Timeseries  SampleTime=Ts
  threshold_coil : ToFile  Filename=C:\Users\papad\Dropbox\Complete_building_simulator_Observers\Results_fz4_sensor\Th_c_C_Gym.mat  SaveFormat=Timeseries  SampleTime=Ts
  threshold_hcoil : ToFile  Filename=C:\Users\papad\Dropbox\Complete_building_simulator_Observers\Results_fz4_sensor\Th_c_H_Gym.mat  SaveFormat=Timeseries  SampleTime=Ts
  threshold_sa : ToFile  Filename=C:\Users\papad\Dropbox\Complete_building_simulator_Observers\Results_fz4_sensor\Th_saC_Gym.mat  SaveFormat=Timeseries  SampleTime=Ts
  threshold_zone : ToFile  Filename=C:\Users\papad\Dropbox\Complete_building_simulator_Observers\Results_fz4_sensor\Th_zC_Gym.mat  SaveFormat=Timeseries  SampleTime=Ts
  Dz : Outport
  wires:
  Demux/8 -> [TzNeighbor]
  Demux/5 -> [Tsa]
  Demux/2 -> [Tamb]
  Demux/6 -> [Tst_c]
  In1/1 -> Demux/1
  Demux/1 -> [Tz]
  Demux/3 -> [Tcc]
  Demux/9 -> Saturation/1
  Sum1/1 -> Unit Delay8/1
  Saturation/1 -> [mcc]
  Unit Delay8/1 -> Tsahat_mat/1, [Tsahat]
  Product11/1 -> Sum1/6
  Dot Product4/1 -> Subtract4/2
  Threshold_sa/1(Out1) -> If2/2, threshold_sa/1  "thr"
  Abs1/1 -> If2/1, residual_sa/1  "res"
  If2/1 -> If Action Subsystem4/Action
  If2/2 -> If Action Subsystem3/Action
  Constant3/1 -> Enabled Subsystem1/1(In1), If Action Subsystem4/1(In1)
  If Action Subsystem4/1(Out1) -> Merge1/1
  If Action Subsystem3/1(Out1) -> Merge1/2
  Constant4/1 -> If Action Subsystem3/1(In1)
  Enabled Subsystem1/1(Out1) -> [Dsai], Detection_sa/1
  Merge1/1 -> Enabled Subsystem1/Enable
  [TzNeighbor] -> Mux4/3
  Mux4/1 -> Dot Product3/1
  [Tamb] -> Mux4/4
  ZoneGains/1 -> Dot Product3/2
  [Tzhat] -> Mux4/1
  Unit Delay9/1 -> Tzhat_mat/1, [Tzhat]
  [Tsa] -> Mux4/2
  Threshold_zone/1(Out1) -> If1/2, threshold_zone/1  "thr"
  Dot Product3/1 -> Unit Delay9/1
  Constant/1 -> Enabled Subsystem2/1(In1), If Action Subsystem2/1(In1)
  Subtract7/1 -> Abs/1, Mux4/5
  Abs/1 -> If1/1, residual_zone/1  "res"
  If1/1 -> If Action Subsystem2/Action
  [Tz] -> Subtract7/1
  [Tzhat] -> Subtract7/2
  If Action Subsystem2/1(Out1) -> Merge/1
  If1/2 -> If Action Subsystem1/Action
  If Action Subsystem1/1(Out1) -> Merge/2
  Constant1/1 -> If Action Subsystem1/1(In1)
  Enabled Subsystem2/1(Out1) -> Dz/1, [Dzi], Detection_zone/1
  Merge/1 -> Enabled Subsystem2/Enable
  Sum/1 -> Unit Delay7/1
  Unit Delay7/1 -> Tchat_mat/1, [Tcchat]
  Abs2/1 -> residual_coil/1, If3/1  "res"
  Constant5/1 -> Enabled Subsystem/1(In1), If Action Subsystem6/1(In1)
  Dot Product16/1 -> Subtract9/2
  [mcc] -> Threshold_coil/1(mc)
  If3/2 -> If Action Subsystem5/Action
  Threshold_coil/1(Out1) -> If3/2, threshold_coil/1  "thr"
  Constant6/1 -> If Action Subsystem5/1(In1)
  If Action Subsystem6/1(Out1) -> Merge2/1
  Subtract9/1 -> Abs2/1, Product5/2
  If3/1 -> If Action Subsystem6/Action
  Enabled Subsystem/1(Out1) -> [Dci], Detection_coil/1
  If Action Subsystem5/1(Out1) -> Merge2/2
  Merge2/1 -> Enabled Subsystem/Enable
  [Tsahat] -> Dot Product4/2
  [Tsa] -> Subtract4/1
  Product8/1 -> Sum1/3
  Product7/1 -> Sum1/2
  Product9/1 -> Sum1/4
  Product6/1 -> Sum1/1
  Product10/1 -> Sum1/5
  Csa/1 -> Dot Product4/1
  Subtract5/1 -> Dot Product12/2
  [mcc] -> Dot Product12/1
  [Tcc] -> Subtract5/2
  [Tst_c] -> Subtract5/1
  [Tcc] -> Subtract9/1
  Product1/1 -> Sum/2
  Product3/1 -> Sum/4
  Product/1 -> Sum/1
  Product5/1 -> Sum/6
  Cc_C/1 -> Dot Product16/1
  [Tcchat] -> Dot Product16/2
  Dot Product12/1 -> Mux3/2
  Constant2/1 -> Mux3/1
  Product2/1 -> Sum/3
  [Tcchat] -> Product/2
  CoilGains/1 -> Product/1
  Mux3/1 -> Product1/2
  CoilGains1/1 -> Product1/1
  Constant8/1 -> Product2/2
  CoilGains2/1 -> Product2/1
  CoilGains3/1 -> Product3/1
  [Tamb] -> Product3/2
  CoilGains5/1 -> Product5/1
  Product4/1 -> Sum/5
  CoilGains4/1 -> Product4/1
  [Tz] -> Product4/2
  [Tsahat] -> Product6/2
  SupplyGains/1 -> Product6/1
  SupplyGains1/1 -> Product7/1
  Constant7/1 -> Product8/2
  SupplyGains2/1 -> Product8/1
  [Tamb] -> Product9/2
  SupplyGains3/1 -> Product9/1
  [Tz] -> Product10/2
  SupplyGains4/1 -> Product10/1
  Subtract4/1 -> Abs1/1, Product11/2
  SupplyGains5/1 -> Product11/1
  Demux/4 -> [Thc]
  Saturation1/1 -> [mhc]
  Demux/10 -> Saturation1/1
  Demux/7 -> [Tst_h]
  [Tcc] -> Mux/1
  [Thc] -> Mux/2
  Mux/1 -> Product7/2
  Enabled Subsystem3/1(Out1) -> Detection_hcoil/1
  Merge3/1 -> Enabled Subsystem3/Enable
  If Action Subsystem7/1(Out1) -> Merge3/2
  If Action Subsystem8/1(Out1) -> Merge3/1
  Unit Delay1/1 -> Thchat_mat/1, [Thchat]
  Sum2/1 -> Unit Delay1/1
  Subtract10/1 -> Abs3/1, Product17/2
  Abs3/1 -> residual_hcoil/1, If4/1
  Dot Product2/1 -> Subtract10/2
  [mhc] -> Threshold_hcoil/1(mhc)
  Threshold_hcoil/1(Out1) -> If4/2, threshold_hcoil/1  "thr"
  If4/1 -> If Action Subsystem8/Action
  If4/2 -> If Action Subsystem7/Action
  Constant10/1 -> Enabled Subsystem3/1(In1), If Action Subsystem8/1(In1)
  Constant11/1 -> If Action Subsystem7/1(In1)
  [mhc] -> Dot Product1/1
  [Thc] -> Subtract8/2
  [Tst_h] -> Subtract8/1
  [Thc] -> Subtract10/1
  Product13/1 -> Sum2/2
  Product15/1 -> Sum2/5
  Product12/1 -> Sum2/1
  Product17/1 -> Sum2/7
  Hc_H/1 -> Dot Product2/1
  [Thchat] -> Dot Product2/2
  Product14/1 -> Sum2/4
  [Thchat] -> Product12/2
  HCoilGains/1 -> Product12/1
  Product18/1 -> Product13/2
  HCoilGains1/1 -> Product13/1
  Constant12/1 -> Product14/2
  HCoilGains2/1 -> Product14/1
  HCoilGains3/1 -> Product15/1
  [Tamb] -> Product15/2
  HCoilGains5/1 -> Product17/1
  Product16/1 -> Sum2/6
  HCoilGains4/1 -> Product16/1
  [Tz] -> Product16/2
  Subtract8/1 -> Dot Product1/2
  Dot Product1/1 -> Product18/2
  Hc_H1/1 -> Product18/1
  [mcc] -> Dot Product5/1
  [Tcc] -> Subtract1/2
  [Tst_c] -> Subtract1/1
  Subtract1/1 -> Dot Product5/2
  Dot Product5/1 -> Product19/2
  Hc_H2/1 -> Product19/1
  Product20/1 -> Sum2/3
  Product19/1 -> Product20/2
  HCoilGains6/1 -> Product20/1
  [mcc] -> Threshold_hcoil/2(mcc)
